simulink library "MOST_Lib"  (R2020b)
settings: Solver=VariableStepAuto  StartTime=0.0  StopTime=10.0  RelTol=1e-3
undefined symbols (not defined in the file or in its folder): simu, wind, windTurbine, windturbine, X, Y, Z
libraries: sm_lib x34, nesl_utility x28, $bdroot x6, simulink_extras x5, simulink x4, WECSim_Lib_Body_Elements x1
(part 1 of 2)
settings Model: VariantFading=off  Creator=kmruehl  LastModifiedBy=emili

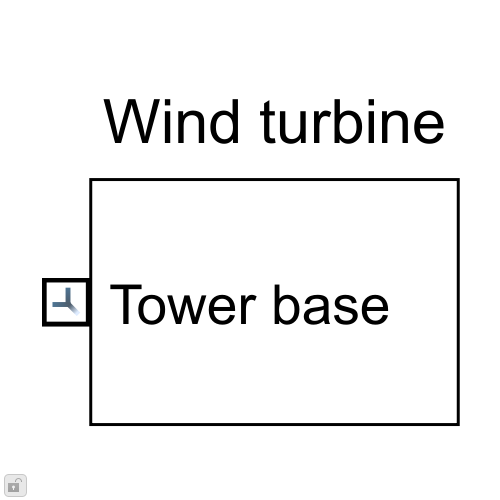
[thumbnail: Simulink's stored preview of the top-level diagram]
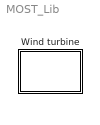
== system MOST_Lib ==
  Wind turbine : Subsystem  VariantControl=sv_1_IEA15MW  mask{windTurbine=windTurbine(1)}
      mask prompts: windTurbine "Wind Number:"
      mask init:
      | h1=[gcb '/Tower/Body Properties'];
      | geomFile=windTurbine.geometryFileTower;
      | set_param(h1,'ExtGeomFileName',geomFile);
      | geomType='STEP';
      | 
      | h1=[gcb '/Nacelle/Body Properties'];
      | geomFile=windTurbine.geometryFileNacelle;
      | set_param(h1,'ExtGeomFileName',geomFile);
      | geomType='STEP';
      | 
      | h1=[gcb '/Hub/Body Properties'];
      | geomFile=windTurbine.geometryFileHub;
      | set_param(h1,'ExtGeomFileName',geomFile);
      | geomType='STEP';
      | 
      | % h1=[gcb '/blade 1/Blade/Body Properties'];
      | % geomFile=windTurbine.geometryFileBlade;
      | % set_param(h1,'ExtGeomFileName',geomFile);
      | % geomType='STEP';
      | 
      | % h1=[gcb '/blade 2/Blade/Body Properties'];
      | % geomFile=windTurbine.geometryFileBlade;
      | % set_param(h1,'ExtGeomFileName',geomFile);
      | % geomType='STEP';
      | % 
      | % h1=[gcb '/blade 3/Blade/Body Properties'];
      | % geomFile=windTurbine.geometryFileBlade;
      | % set_param(h1,'ExtGeomFileName',geomFile);
      | % geomType='STEP';
      | 
      | % toWorkSpace: outputs
      | h=[gcb '/SignalOutput/To Workspace'];
      | responseName=['windTurbine' num2str(windTurbine.number) '_out'];
      | set_param(h,'VariableName',responseName);

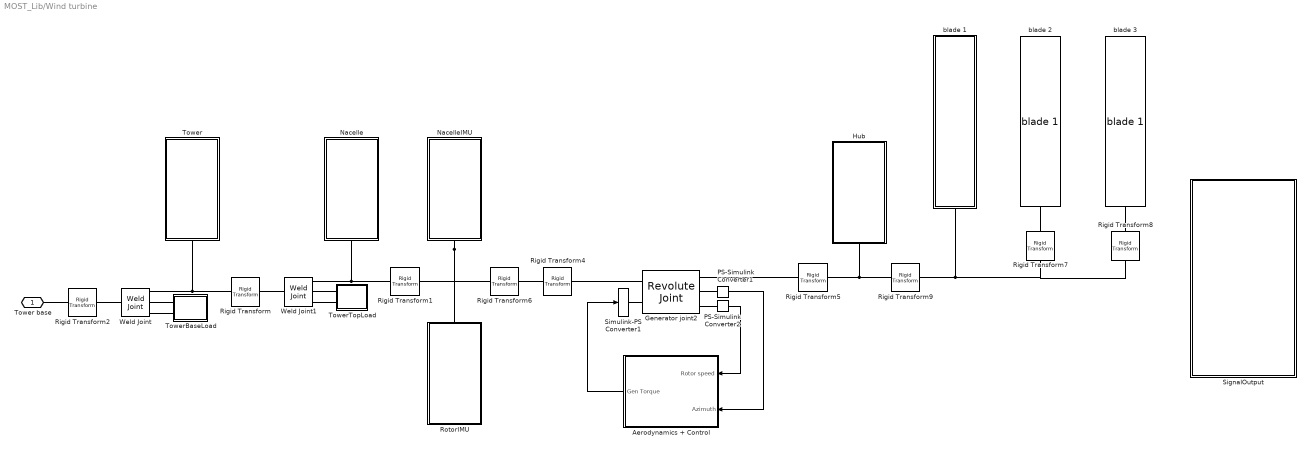
== system MOST_Lib/Wind turbine ==
  Aerodynamics + Control : Subsystem  VariantControl=sv_control2
  Generator joint2 : sm_lib/Joints/Revolute Joint  JointMode=Normal  SenseConstraintForce=off  SenseConstraintForceX=off  SenseConstraintForceY=off  SenseConstraintForceZ=off  SenseConstraintTorque=off  SenseConstraintTorqueX=off  SenseConstraintTorqueY=off  SenseConstraintTorqueZ=off  SenseTotalForce=off  SenseTotalForceX=off  SenseTotalForceY=off  SenseTotalForceZ=off  SenseTotalTorque=off  SenseTotalTorqueX=off  SenseTotalTorqueY=off  SenseTotalTorqueZ=off  CompositeWrenchDir=FollowerOnBase  CompositeWrenchFrame=BaseFrame  EquilibriumPosition=0  EquilibriumPositionUnits=deg  SpringStiffness=0  SpringStiffnessUnits=N*m/deg  DampingCoefficient=0  DampingCoefficientUnits=N*m/(deg/s)  TorqueActuationMode=InputTorque  MotionActuationMode=ComputedMotion  SensePosition=on  SenseVelocity=on  SenseAcceleration=off  SenseTorqueForce=off  SenseLowerLimitTorqueForce=off  SenseUpperLimitTorqueForce=off  LowerLimitSpecify=off  LowerLimitBound=-90  LowerLimitBoundUnits=deg  LowerLimitStiffness=1e4  LowerLimitStiffnessUnits=N*m/deg  LowerLimitDamping=10  LowerLimitDampingUnits=N*m/(deg/s)  LowerLimitTransitionRegionWidth=0.1  LowerLimitTransitionRegionWidthUnits=deg  UpperLimitSpecify=off  UpperLimitBound=90  UpperLimitBoundUnits=deg  UpperLimitStiffness=1e4  UpperLimitStiffnessUnits=N*m/deg  UpperLimitDamping=10  UpperLimitDampingUnits=N*m/(deg/s)  UpperLimitTransitionRegionWidth=0.1  UpperLimitTransitionRegionWidthUnits=deg  PositionTargetSpecify=on  PositionTargetPriority=High  PositionTargetValue=0  PositionTargetValueUnits=deg  VelocityTargetSpecify=on  VelocityTargetPriority=High  VelocityTargetValue=windTurbine.omega0  VelocityTargetValueUnits=rad/s
  Hub : Subsystem
  Nacelle : Subsystem
  NacelleIMU : Subsystem
  PS-Simulink Converter1 : nesl_utility/PS-Simulink Converter  PhysicalDomain=network_engine_domain  SubClassName=ps_output  LeftPortType=input  RightPortType=output  PseudoPeriodic=off  Frequencies=[]  VectorFormat=1-D array  Unit=rad  AffineConversion=off
  PS-Simulink Converter2 : nesl_utility/PS-Simulink Converter  PhysicalDomain=network_engine_domain  SubClassName=ps_output  LeftPortType=input  RightPortType=output  PseudoPeriodic=off  Frequencies=[]  VectorFormat=1-D array  Unit=rad/s  AffineConversion=off
  Rigid Transform : sm_lib/Frames and Transforms/Rigid Transform  TranslationMethod=StandardAxis  TranslationStandardOffset=windTurbine.tower.height  TranslationLengthUnit=m  TranslationStandardAxis=+Z  TranslationCartesianOffset=[0 0 0]  TranslationROffset=0  TranslationROffsetUnits=m  TranslationZOffset=0  TranslationZOffsetUnits=m  TranslationThetaOffset=0  TranslationThetaOffsetUnits=deg  RotationMethod=None  RotationAngleUnits=deg  RotationStandardAxis=+Z  RotationAngle=0.0  RotationArbitraryAxis=[0 0 1]  FollAlignAxisA=+X  BaseAlignAxisA=+Y  FollAlignAxisB=+Y  BaseAlignAxisB=+Z  RotationSequenceAxes=FollowerAxes  RotationSequence=XYX  RotationSequenceAngles=[0 0 0]  RotationSequenceAnglesUnits=deg  RotationMatrix=[1 0 0; 0 1 0; 0 0 1]  Quaternion=[1 0 0 0]
  Rigid Transform1 : sm_lib/Frames and Transforms/Rigid Transform  TranslationMethod=StandardAxis  TranslationStandardOffset=windTurbine.nacelle.Twr2Shft  TranslationLengthUnit=m  TranslationStandardAxis=+Z  TranslationCartesianOffset=[0 0 0]  TranslationROffset=0  TranslationROffsetUnits=m  TranslationZOffset=0  TranslationZOffsetUnits=m  TranslationThetaOffset=0  TranslationThetaOffsetUnits=deg  RotationMethod=None  RotationAngleUnits=deg  RotationStandardAxis=+Z  RotationAngle=0.0  RotationArbitraryAxis=[0 0 1]  FollAlignAxisA=+X  BaseAlignAxisA=+Y  FollAlignAxisB=+Y  BaseAlignAxisB=+Z  RotationSequenceAxes=FollowerAxes  RotationSequence=XYX  RotationSequenceAngles=[0 0 0]  RotationSequenceAnglesUnits=deg  RotationMatrix=[1 0 0; 0 1 0; 0 0 1]  Quaternion=[1 0 0 0]
  Rigid Transform2 : sm_lib/Frames and Transforms/Rigid Transform  TranslationMethod=StandardAxis  TranslationStandardOffset=windTurbine.tower.offset  TranslationLengthUnit=m  TranslationStandardAxis=+Z  TranslationCartesianOffset=[0 0 0]  TranslationROffset=0  TranslationROffsetUnits=m  TranslationZOffset=0  TranslationZOffsetUnits=m  TranslationThetaOffset=0  TranslationThetaOffsetUnits=deg  RotationMethod=None  RotationAngleUnits=deg  RotationStandardAxis=+Z  RotationAngle=0.0  RotationArbitraryAxis=[0 0 1]  FollAlignAxisA=+X  BaseAlignAxisA=+Y  FollAlignAxisB=+Y  BaseAlignAxisB=+Z  RotationSequenceAxes=FollowerAxes  RotationSequence=XYX  RotationSequenceAngles=[0 0 0]  RotationSequenceAnglesUnits=deg  RotationMatrix=[1 0 0; 0 1 0; 0 0 1]  Quaternion=[1 0 0 0]
  Rigid Transform4 : sm_lib/Frames and Transforms/Rigid Transform  TranslationMethod=None  TranslationStandardOffset=0  TranslationLengthUnit=m  TranslationStandardAxis=+Z  TranslationCartesianOffset=[0 0 0]  TranslationROffset=0  TranslationROffsetUnits=m  TranslationZOffset=0  TranslationZOffsetUnits=m  TranslationThetaOffset=0  TranslationThetaOffsetUnits=deg  RotationMethod=StandardAxis  RotationAngleUnits=deg  RotationStandardAxis=+Y  RotationAngle=90  RotationArbitraryAxis=[0 0 1]  FollAlignAxisA=+X  BaseAlignAxisA=+Y  FollAlignAxisB=+Y  BaseAlignAxisB=+Z  RotationSequenceAxes=FollowerAxes  RotationSequence=XYX  RotationSequenceAngles=[0 0 0]  RotationSequenceAnglesUnits=deg  RotationMatrix=[1 0 0; 0 1 0; 0 0 1]  Quaternion=[1 0 0 0]
  Rigid Transform5 : sm_lib/Frames and Transforms/Rigid Transform  TranslationMethod=None  TranslationStandardOffset=0  TranslationLengthUnit=m  TranslationStandardAxis=+Z  TranslationCartesianOffset=[0 0 0]  TranslationROffset=0  TranslationROffsetUnits=m  TranslationZOffset=0  TranslationZOffsetUnits=m  TranslationThetaOffset=0  TranslationThetaOffsetUnits=deg  RotationMethod=StandardAxis  RotationAngleUnits=deg  RotationStandardAxis=-Y  RotationAngle=90  RotationArbitraryAxis=[0 0 1]  FollAlignAxisA=+X  BaseAlignAxisA=+Y  FollAlignAxisB=+Y  BaseAlignAxisB=+Z  RotationSequenceAxes=FollowerAxes  RotationSequence=XYX  RotationSequenceAngles=[0 0 0]  RotationSequenceAnglesUnits=deg  RotationMatrix=[1 0 0; 0 1 0; 0 0 1]  Quaternion=[1 0 0 0]
  Rigid Transform6 : sm_lib/Frames and Transforms/Rigid Transform  TranslationMethod=None  TranslationStandardOffset=0  TranslationLengthUnit=m  TranslationStandardAxis=+Z  TranslationCartesianOffset=[0 0 0]  TranslationROffset=0  TranslationROffsetUnits=m  TranslationZOffset=0  TranslationZOffsetUnits=m  TranslationThetaOffset=0  TranslationThetaOffsetUnits=deg  RotationMethod=StandardAxis  RotationAngleUnits=deg  RotationStandardAxis=+Y  RotationAngle=windTurbine.nacelle.tiltangle  RotationArbitraryAxis=[0 0 1]  FollAlignAxisA=+X  BaseAlignAxisA=+Y  FollAlignAxisB=+Y  BaseAlignAxisB=+Z  RotationSequenceAxes=FollowerAxes  RotationSequence=XYX  RotationSequenceAngles=[0 0 0]  RotationSequenceAnglesUnits=deg  RotationMatrix=[1 0 0; 0 1 0; 0 0 1]  Quaternion=[1 0 0 0]
  Rigid Transform7 : sm_lib/Frames and Transforms/Rigid Transform  TranslationMethod=None  TranslationStandardOffset=0  TranslationLengthUnit=m  TranslationStandardAxis=+Z  TranslationCartesianOffset=[0 0 0]  TranslationROffset=0  TranslationROffsetUnits=m  TranslationZOffset=0  TranslationZOffsetUnits=m  TranslationThetaOffset=0  TranslationThetaOffsetUnits=deg  RotationMethod=StandardAxis  RotationAngleUnits=deg  RotationStandardAxis=+X  RotationAngle=120  RotationArbitraryAxis=[0 0 1]  FollAlignAxisA=+X  BaseAlignAxisA=+Y  FollAlignAxisB=+Y  BaseAlignAxisB=+Z  RotationSequenceAxes=FollowerAxes  RotationSequence=XYX  RotationSequenceAngles=[0 0 0]  RotationSequenceAnglesUnits=deg  RotationMatrix=[1 0 0; 0 1 0; 0 0 1]  Quaternion=[1 0 0 0]
  Rigid Transform8 : sm_lib/Frames and Transforms/Rigid Transform  TranslationMethod=None  TranslationStandardOffset=0  TranslationLengthUnit=m  TranslationStandardAxis=+Z  TranslationCartesianOffset=[0 0 0]  TranslationROffset=0  TranslationROffsetUnits=m  TranslationZOffset=0  TranslationZOffsetUnits=m  TranslationThetaOffset=0  TranslationThetaOffsetUnits=deg  RotationMethod=StandardAxis  RotationAngleUnits=deg  RotationStandardAxis=+X  RotationAngle=240  RotationArbitraryAxis=[0 0 1]  FollAlignAxisA=+X  BaseAlignAxisA=+Y  FollAlignAxisB=+Y  BaseAlignAxisB=+Z  RotationSequenceAxes=FollowerAxes  RotationSequence=XYX  RotationSequenceAngles=[0 0 0]  RotationSequenceAnglesUnits=deg  RotationMatrix=[1 0 0; 0 1 0; 0 0 1]  Quaternion=[1 0 0 0]
  Rigid Transform9 : sm_lib/Frames and Transforms/Rigid Transform  TranslationMethod=StandardAxis  TranslationStandardOffset=windTurbine.hub.overhang  TranslationLengthUnit=m  TranslationStandardAxis=+X  TranslationCartesianOffset=[0 0 0]  TranslationROffset=0  TranslationROffsetUnits=m  TranslationZOffset=0  TranslationZOffsetUnits=m  TranslationThetaOffset=0  TranslationThetaOffsetUnits=deg  RotationMethod=None  RotationAngleUnits=deg  RotationStandardAxis=+Z  RotationAngle=0.0  RotationArbitraryAxis=[0 0 1]  FollAlignAxisA=+X  BaseAlignAxisA=+Y  FollAlignAxisB=+Y  BaseAlignAxisB=+Z  RotationSequenceAxes=FollowerAxes  RotationSequence=XYX  RotationSequenceAngles=[0 0 0]  RotationSequenceAnglesUnits=deg  RotationMatrix=[1 0 0; 0 1 0; 0 0 1]  Quaternion=[1 0 0 0]
  RotorIMU : Subsystem
  SignalOutput : Subsystem
  Simulink-PS Converter1 : nesl_utility/Simulink-PS Converter  PhysicalDomain=network_engine_domain  SubClassName=ps_input  LeftPortType=input  RightPortType=output  PseudoPeriodic=off  Frequencies=[]  NoiseDistribution=none  NoiseParameters=[]  Unit=N*m  AffineConversion=off  FilteringAndDerivatives=provide  SimscapeFilterOrder=2  InputFilterTimeConstant=0.1  UdotUserProvided=0
  Tower : Subsystem
  TowerBaseLoad : Subsystem
  TowerTopLoad : Subsystem
  Weld Joint : sm_lib/Joints/Weld Joint  JointMode=Normal  SenseConstraintForce=on  SenseConstraintForceX=off  SenseConstraintForceY=off  SenseConstraintForceZ=off  SenseConstraintTorque=on  SenseConstraintTorqueX=off  SenseConstraintTorqueY=off  SenseConstraintTorqueZ=off  SenseTotalForce=off  SenseTotalForceX=off  SenseTotalForceY=off  SenseTotalForceZ=off  SenseTotalTorque=off  SenseTotalTorqueX=off  SenseTotalTorqueY=off  SenseTotalTorqueZ=off  CompositeWrenchDir=FollowerOnBase  CompositeWrenchFrame=BaseFrame
  Weld Joint1 : sm_lib/Joints/Weld Joint  JointMode=Normal  SenseConstraintForce=on  SenseConstraintForceX=off  SenseConstraintForceY=off  SenseConstraintForceZ=off  SenseConstraintTorque=on  SenseConstraintTorqueX=off  SenseConstraintTorqueY=off  SenseConstraintTorqueZ=off  SenseTotalForce=off  SenseTotalForceX=off  SenseTotalForceY=off  SenseTotalForceZ=off  SenseTotalTorque=off  SenseTotalTorqueX=off  SenseTotalTorqueY=off  SenseTotalTorqueZ=off  CompositeWrenchDir=FollowerOnBase  CompositeWrenchFrame=BaseFrame
  blade 1 : Subsystem  mask{bladeNumber=1, windTurbine=windTurbine}
      mask prompts: bladeNumber "Blade Number:"; windTurbine "windTurbine Number:"
      mask init:
      | h=[gcb '/bladeRootLoad/Goto'];
      |     responseName=['blade' num2str(bladeNumber) 'RootLoad'];
      |     set_param(h,'GotoTag',responseName);
      | 
      |     if exist('windTurbine','var') && ~isempty(windTurbine)
      |         h1=[gcb '/Blade/Body Properties'];
      |         geomFile=windTurbine.geometryFileBlade;
      |         set_param(h1,'ExtGeomFileName',geomFile);
      |     
      |         % mass=windTurbine.blade.mass;
      |         % set_param(h1,'Mass',mass)
      |         % 
      |         % inertia=windTurbine.blade.inertia;
      |         % set_param(h1,'MomentsOfInertia',inertia)
      |     end
  blade 2 : $bdroot/Wind turbine/blade 1  bladeNumber=2
  blade 3 : $bdroot/Wind turbine/blade 1  bladeNumber=3
  Tower base : PMIOPort  Side=Right
  wires:
  Aerodynamics + Control/1(Gen Torque) -> Simulink-PS Converter1/1
  PS-Simulink Converter1/1 -> Aerodynamics + Control/2(Azimuth)
  PS-Simulink Converter2/1 -> Aerodynamics + Control/1(" Rotor speed")
  connections:
  Nacelle/LConn1 -- Rigid Transform1/LConn1 -- Weld Joint1/RConn1
  blade 2/LConn1 -- Rigid Transform7/RConn1
  NacelleIMU/LConn1 -- Rigid Transform1/RConn1 -- RotorIMU/LConn1 -- Rigid Transform6/LConn1
  Hub/LConn1 -- Rigid Transform9/LConn1 -- Rigid Transform5/RConn1
  Tower/LConn1 -- Rigid Transform/LConn1 -- Weld Joint/RConn1
  Rigid Transform6/RConn1 -- Rigid Transform4/LConn1
  Rigid Transform4/RConn1 -- Generator joint2/LConn1
  Rigid Transform/RConn1 -- Weld Joint1/LConn1
  Rigid Transform2/LConn1 -- Tower base/RConn1
  PS-Simulink Converter2/LConn1 -- Generator joint2/RConn3
  Generator joint2/RConn1 -- Rigid Transform5/LConn1
  Rigid Transform2/RConn1 -- Weld Joint/LConn1
  Simulink-PS Converter1/RConn1 -- Generator joint2/LConn2
  PS-Simulink Converter1/LConn1 -- Generator joint2/RConn2
  Rigid Transform9/RConn1 -- Rigid Transform8/LConn1 -- Rigid Transform7/LConn1 -- blade 1/LConn1
  blade 3/LConn1 -- Rigid Transform8/RConn1
  Weld Joint/RConn2 -- TowerBaseLoad/LConn1
  Weld Joint/RConn3 -- TowerBaseLoad/LConn2
  Weld Joint1/RConn2 -- TowerTopLoad/LConn1
  Weld Joint1/RConn3 -- TowerTopLoad/LConn2

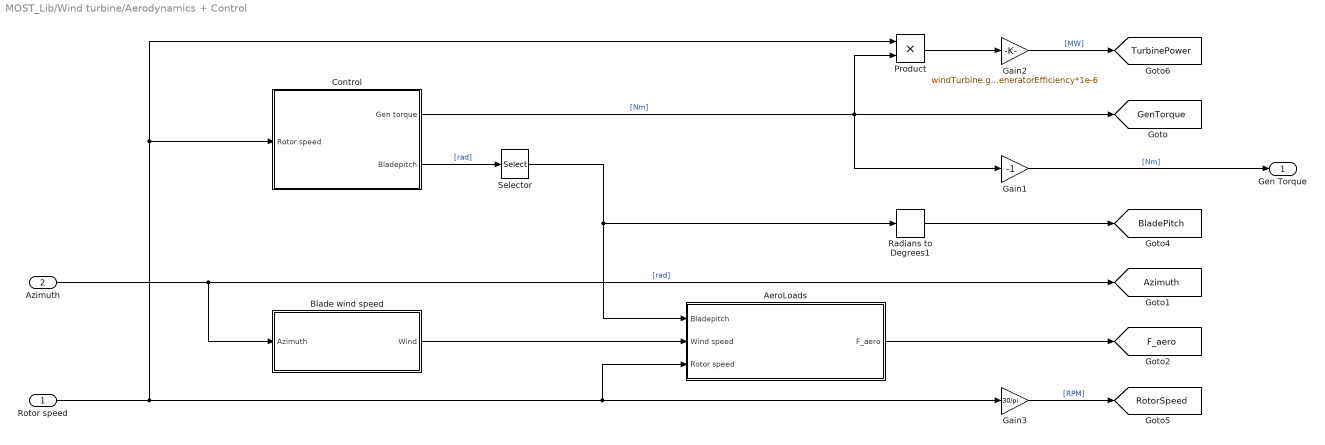
== system MOST_Lib/Wind turbine/Aerodynamics + Control ==
  " Rotor speed" : Inport
  Azimuth : Inport  Port=2
  AeroLoads : Subsystem
  Blade wind speed : Subsystem  Variant=on
  Control : Subsystem  Variant=on  VariantControl=sv_IEA10MW  mask{windturbine=windTurbine, wind=wind(1)}
      mask init:
      | h = [gcb,'/Baseline'];
      | sv1 = ['sv_' num2str(windturbine.number) '_control1'];
      | set_param(h,'VariantControl',sv1);
      | 
      | h = [gcb,'/ROSCO'];
      | sv2 = ['sv_' num2str(windturbine.number) '_control2'];
      | set_param(h,'VariantControl',sv2);
  Gain1 : Gain  Gain=-1
  Gain2 : Gain  Gain=windTurbine.generatorEfficiency*1e-6
  Gain3 : Gain  Gain=30/pi
  Product : Product
  Radians to Degrees1 : simulink_extras/Transformations/Radians to Degrees
  Selector : Selector  InputPortWidth=3  IndexOptions=Index vector (dialog)  Indices=1  OutputSizes=1
  Gen Torque : Outport  VectorParamsAs1DForOutWhenUnconnected=off
  tag scope: Azimuth=global, BladePitch=global, F_aero=global, GenTorque=global, RotorSpeed=global, TurbinePower=global
  wires:
  " Rotor speed"/1 -> Gain3/1, AeroLoads/3(Rotor speed), Product/1, Control/1(Rotor speed)
  Control/2(Bladepitch) -> Selector/1  "[rad]"
  Control/1(Gen torque) -> Product/2, Gain1/1, [GenTorque]  "[Nm]"
  Product/1 -> Gain2/1
  Azimuth/1 -> Blade wind speed/1(Azimuth), [Azimuth]  "[rad]"
  Gain3/1 -> [RotorSpeed]  "[RPM]"
  Gain1/1 -> Gen Torque/1  "[Nm]"
  Gain2/1 -> [TurbinePower]  "[MW]"
  Blade wind speed/1(Wind) -> AeroLoads/2(Wind speed)
  AeroLoads/1(F_aero) -> [F_aero]
  Radians to Degrees1/1 -> [BladePitch]
  Selector/1 -> AeroLoads/1(Bladepitch), Radians to Degrees1/1

== system MOST_Lib/Wind turbine/Hub ==
  Body Properties : sm_lib/Body Elements/File Solid  ExtGeomFileName=geometry\IEA15MW_Hub.STEP  UnitType=Custom  ExtGeomFileUnits=m  StepReaderType=HEX  ExportConvexHullGeometry=off  InertiaType=Custom  Mass=windTurbine.hub.mass  MassUnits=kg  CenterOfMass=[0 0 0]  CenterOfMassUnits=m  MomentsOfInertia=windTurbine.hub.inertia  MomentsOfInertiaUnits=kg*m^2  ProductsOfInertia=windTurbine.hub.inertiaProducts  ProductsOfInertiaUnits=kg*m^2  BasedOnType=Density  Density=1000  DensityUnits=kg/(m^3)  DoExposeReferenceFrame=on  SerializedFrames=<Frames/>  GraphicType=FromGeometry  MarkerShape=Sphere  MarkerSize=10  MarkerSizeUnits=m  GraphicVisPropType=AdvancedVisualProperties  GraphicDiffuseColor=[1 1 1]  GraphicSpecularColor=[0.5 0.5 0.5 1.0]  GraphicAmbientColor=[0.15 0.15 0.15 1.0]  GraphicEmissiveColor=[0.0 0.0 0.0 1.0]  GraphicShininess=75  GraphicOpacity=1
  Constant : Constant  Value=-windTurbine.hub.mass*simu.gravity
  External Force and Torque : sm_lib/Forces and Torques/External Force and Torque  ForceResolutionFrame=World  EnableForceX=off  EnableForceY=off  EnableForceZ=on  EnableForce=off  TorqueResolutionFrame=AttachedFrame  EnableTorqueX=off  EnableTorqueY=off  EnableTorqueZ=off  EnableTorque=off
  Rigid Transform (Setup CG) : sm_lib/Frames and Transforms/Rigid Transform  TranslationMethod=StandardAxis  TranslationStandardOffset=windTurbine.hub.overhang  TranslationLengthUnit=m  TranslationStandardAxis=+X  TranslationCartesianOffset=windTurbine.hub.overhang  TranslationROffset=0  TranslationROffsetUnits=m  TranslationZOffset=0  TranslationZOffsetUnits=m  TranslationThetaOffset=0  TranslationThetaOffsetUnits=deg  RotationMethod=None  RotationAngleUnits=rad  RotationStandardAxis=+Y  RotationAngle=0.05  RotationArbitraryAxis=[0.57735026918962584 0.57735026918962584 0.57735026918962584]  FollAlignAxisA=+X  BaseAlignAxisA=+Y  FollAlignAxisB=+Y  BaseAlignAxisB=+Z  RotationSequenceAxes=FollowerAxes  RotationSequence=XYX  RotationSequenceAngles=[0 0 0]  RotationSequenceAnglesUnits=deg  RotationMatrix=[1 0 0; 0 1 0; 0 0 1]  Quaternion=[1 0 0 0]
  Simulink-PS Converter : nesl_utility/Simulink-PS Converter  PhysicalDomain=network_engine_domain  SubClassName=ps_input  LeftPortType=input  RightPortType=output  PseudoPeriodic=off  Frequencies=[]  NoiseDistribution=none  NoiseParameters=[]  Unit=1  AffineConversion=off  FilteringAndDerivatives=provide  SimscapeFilterOrder=1  InputFilterTimeConstant=0.001  UdotUserProvided=0
  Conn1 : PMIOPort  Side=Left
  wires:
  Constant/1 -> Simulink-PS Converter/1
  connections:
  Rigid Transform (Setup CG)/RConn1 -- Body Properties/RConn1 -- External Force and Torque/RConn1
  Rigid Transform (Setup CG)/LConn1 -- Conn1/RConn1
  Simulink-PS Converter/RConn1 -- External Force and Torque/LConn1

== system MOST_Lib/Wind turbine/Nacelle ==
  Body Properties : sm_lib/Body Elements/File Solid  ExtGeomFileName=geometry\IEA15MW_Nacelle.STEP  UnitType=Custom  ExtGeomFileUnits=m  StepReaderType=HEX  ExportConvexHullGeometry=off  InertiaType=Custom  Mass=windTurbine.nacelle.mass  MassUnits=kg  CenterOfMass=[0 0 0]  CenterOfMassUnits=m  MomentsOfInertia=windTurbine.nacelle.inertia  MomentsOfInertiaUnits=kg*m^2  ProductsOfInertia=windTurbine.nacelle.inertiaProducts  ProductsOfInertiaUnits=kg*m^2  BasedOnType=Density  Density=1000  DensityUnits=kg/(m^3)  DoExposeReferenceFrame=on  SerializedFrames=<Frames/>  GraphicType=FromGeometry  MarkerShape=Sphere  MarkerSize=10  MarkerSizeUnits=m  GraphicVisPropType=SimpleVisualProperties  GraphicDiffuseColor=[1 1 1]  GraphicSpecularColor=[0.5 0.5 0.5 1.0]  GraphicAmbientColor=[0.15 0.15 0.15 1.0]  GraphicEmissiveColor=[0.0 0.0 0.0 1.0]  GraphicShininess=75  GraphicOpacity=1
  Constant : Constant  Value=-windTurbine.nacelle.mass*simu.gravity
  Constant1 : Constant  Value=-windTurbine.nacelle.mass_yawBearing*simu.gravity
  External Force and Torque : sm_lib/Forces and Torques/External Force and Torque  ForceResolutionFrame=World  EnableForceX=off  EnableForceY=off  EnableForceZ=on  EnableForce=off  TorqueResolutionFrame=AttachedFrame  EnableTorqueX=off  EnableTorqueY=off  EnableTorqueZ=off  EnableTorque=off
  External Force and Torque1 : sm_lib/Forces and Torques/External Force and Torque  ForceResolutionFrame=World  EnableForceX=off  EnableForceY=off  EnableForceZ=on  EnableForce=off  TorqueResolutionFrame=AttachedFrame  EnableTorqueX=off  EnableTorqueY=off  EnableTorqueZ=off  EnableTorque=off
  Inertia : sm_lib/Body Elements/Inertia  InertiaType=PointMass  Mass=windTurbine.nacelle.mass_yawBearing  MassUnits=kg  CenterOfMass=[0 0 0]  CenterOfMassUnits=m  MomentsOfInertia=[1 1 1]  MomentsOfInertiaUnits=kg*m^2  ProductsOfInertia=[0 0 0]  ProductsOfInertiaUnits=kg*m^2  GraphicType=Marker  MarkerShape=Inertia  MarkerSize=20  GraphicVisPropType=SimpleVisualProperties  GraphicDiffuseColor=[0.5 0.5 0.5]  GraphicSpecularColor=[0.5 0.5 0.5 1.0]  GraphicAmbientColor=[0.15 0.15 0.15 1.0]  GraphicEmissiveColor=[0.0 0.0 0.0 1.0]  GraphicShininess=75  GraphicOpacity=1.0
  Rigid Transform (Setup CG) : sm_lib/Frames and Transforms/Rigid Transform  TranslationMethod=Cartesian  TranslationStandardOffset=0  TranslationLengthUnit=m  TranslationStandardAxis=+Z  TranslationCartesianOffset=windTurbine.nacelle.cog_rel  TranslationROffset=0  TranslationROffsetUnits=m  TranslationZOffset=0  TranslationZOffsetUnits=m  TranslationThetaOffset=0  TranslationThetaOffsetUnits=deg  RotationMethod=None  RotationAngleUnits=rad  RotationStandardAxis=+Y  RotationAngle=0.05  RotationArbitraryAxis=[0.57735026918962584 0.57735026918962584 0.57735026918962584]  FollAlignAxisA=+X  BaseAlignAxisA=+Y  FollAlignAxisB=+Y  BaseAlignAxisB=+Z  RotationSequenceAxes=FollowerAxes  RotationSequence=XYX  RotationSequenceAngles=[0 0 0]  RotationSequenceAnglesUnits=deg  RotationMatrix=[1 0 0; 0 1 0; 0 0 1]  Quaternion=[1 0 0 0]
  Simulink-PS Converter : nesl_utility/Simulink-PS Converter  PhysicalDomain=network_engine_domain  SubClassName=ps_input  LeftPortType=input  RightPortType=output  PseudoPeriodic=off  Frequencies=[]  NoiseDistribution=none  NoiseParameters=[]  Unit=1  AffineConversion=off  FilteringAndDerivatives=provide  SimscapeFilterOrder=1  InputFilterTimeConstant=0.001  UdotUserProvided=0
  Simulink-PS Converter1 : nesl_utility/Simulink-PS Converter  PhysicalDomain=network_engine_domain  SubClassName=ps_input  LeftPortType=input  RightPortType=output  PseudoPeriodic=off  Frequencies=[]  NoiseDistribution=none  NoiseParameters=[]  Unit=1  AffineConversion=off  FilteringAndDerivatives=provide  SimscapeFilterOrder=1  InputFilterTimeConstant=0.001  UdotUserProvided=0
  Conn1 : PMIOPort  Side=Left
  wires:
  Constant/1 -> Simulink-PS Converter/1
  Constant1/1 -> Simulink-PS Converter1/1
  connections:
  Rigid Transform (Setup CG)/RConn1 -- Body Properties/RConn1 -- External Force and Torque/RConn1
  Inertia/RConn1 -- Rigid Transform (Setup CG)/LConn1 -- Conn1/RConn1 -- External Force and Torque1/RConn1
  Simulink-PS Converter/RConn1 -- External Force and Torque/LConn1
  Simulink-PS Converter1/RConn1 -- External Force and Torque1/LConn1

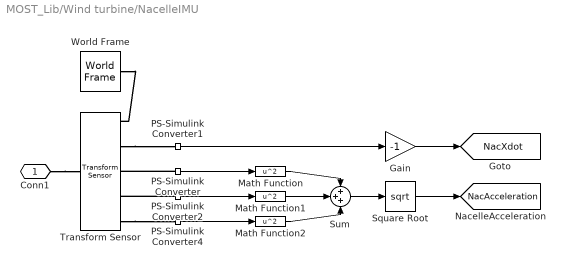
== system MOST_Lib/Wind turbine/NacelleIMU ==
  Gain : Gain  Gain=-1
  Math Function : Math  Operator=square  SaturateOnIntegerOverflow=on
  Math Function1 : Math  Operator=square  SaturateOnIntegerOverflow=on
  Math Function2 : Math  Operator=square  SaturateOnIntegerOverflow=on
  PS-Simulink Converter{,1..2,4} (4 alike) : nesl_utility/PS-Simulink Converter  PhysicalDomain=network_engine_domain  SubClassName=ps_output  LeftPortType=input  RightPortType=output  PseudoPeriodic=off  Frequencies=[]  VectorFormat=1-D array  Unit=inherit  AffineConversion=off
  Square Root : Sqrt  SaturateOnIntegerOverflow=on
  Sum : Sum  Inputs=+++
  Transform Sensor : sm_lib/Frames and Transforms/Transform Sensor  MeasurementFrame=World  SenseAngle=off  SenseAxis=off  SenseQ=off  SenseR=off  SenseRotationSequence=off  RotationSequence=XYX  SenseOmegaX=off  SenseOmegaY=off  SenseOmegaZ=off  SenseQDot=off  SenseRDot=off  SenseAlphaX=off  SenseAlphaY=off  SenseAlphaZ=off  SenseQDDot=off  SenseRDDot=off  SenseX=off  SenseY=off  SenseZ=off  SenseRad=off  SenseAzim=off  SenseDist=off  SenseInc=off  SenseXDot=on  SenseYDot=off  SenseZDot=off  SenseRadDot=off  SenseAzimDot=off  SenseDistDot=off  SenseIncDot=off  SenseXDDot=on  SenseYDDot=on  SenseZDDot=on  SenseRadDDot=off  SenseAzimDDot=off  SenseDistDDot=off  SenseIncDDot=off
  World Frame : sm_lib/Frames and Transforms/World Frame
  Conn1 : PMIOPort  Side=Left
  tag scope: NacAcceleration=global, NacXdot=global
  wires:
  PS-Simulink Converter1/1 -> Gain/1
  PS-Simulink Converter2/1 -> Math Function1/1
  Math Function2/1 -> Sum/3
  Math Function/1 -> Sum/1
  PS-Simulink Converter/1 -> Math Function/1
  Math Function1/1 -> Sum/2
  PS-Simulink Converter4/1 -> Math Function2/1
  Sum/1 -> Square Root/1
  Gain/1 -> [NacXdot]
  Square Root/1 -> [NacAcceleration]
  connections:
  Transform Sensor/RConn5 -- PS-Simulink Converter4/LConn1
  PS-Simulink Converter2/LConn1 -- Transform Sensor/RConn4
  PS-Simulink Converter/LConn1 -- Transform Sensor/RConn3
  Transform Sensor/RConn2 -- PS-Simulink Converter1/LConn1
  Conn1/RConn1 -- Transform Sensor/LConn1
  World Frame/RConn1 -- Transform Sensor/RConn1

== system MOST_Lib/Wind turbine/RotorIMU ==
  PS-Simulink Converter{,1,3..4} (4 alike) : nesl_utility/PS-Simulink Converter  PhysicalDomain=network_engine_domain  SubClassName=ps_output  LeftPortType=input  RightPortType=output  PseudoPeriodic=off  Frequencies=[]  VectorFormat=1-D array  Unit=inherit  AffineConversion=off
  Transform Sensor : sm_lib/Frames and Transforms/Transform Sensor  MeasurementFrame=World  SenseAngle=off  SenseAxis=off  SenseQ=on  SenseR=off  SenseRotationSequence=off  RotationSequence=XYX  SenseOmegaX=off  SenseOmegaY=on  SenseOmegaZ=on  SenseQDot=off  SenseRDot=off  SenseAlphaX=off  SenseAlphaY=off  SenseAlphaZ=off  SenseQDDot=off  SenseRDDot=off  SenseX=off  SenseY=off  SenseZ=off  SenseRad=off  SenseAzim=off  SenseDist=off  SenseInc=off  SenseXDot=on  SenseYDot=off  SenseZDot=off  SenseRadDot=off  SenseAzimDot=off  SenseDistDot=off  SenseIncDot=off  SenseXDDot=off  SenseYDDot=off  SenseZDDot=off  SenseRadDDot=off  SenseAzimDDot=off  SenseDistDDot=off  SenseIncDDot=off
  World Frame : sm_lib/Frames and Transforms/World Frame
  quaternion2EulXYZ : WECSim_Lib_Body_Elements/Rigid Body/Hydrodynamic Body/Structure/Motion Sensor/quaternion2EulXYZ  SystemSampleTime=-1  VariantActivationTime=update diagram  Latency=0  AutoFrameSizeCalculation=off
  Conn1 : PMIOPort  Side=Left
  tag scope: HUBpitch=global, HUBrot=global, HUBvx=global, HUByaw=global
  wires:
  PS-Simulink Converter4/1 -> [HUBvx]
  PS-Simulink Converter1/1 -> quaternion2EulXYZ/1
  PS-Simulink Converter/1 -> [HUBpitch]
  PS-Simulink Converter3/1 -> [HUByaw]
  quaternion2EulXYZ/1 -> [HUBrot]
  connections:
  PS-Simulink Converter1/LConn1 -- Transform Sensor/RConn2
  PS-Simulink Converter/LConn1 -- Transform Sensor/RConn3
  Transform Sensor/RConn4 -- PS-Simulink Converter3/LConn1
  Transform Sensor/RConn5 -- PS-Simulink Converter4/LConn1
  Transform Sensor/RConn1 -- Conn1/RConn1
  Transform Sensor/LConn1 -- World Frame/RConn1

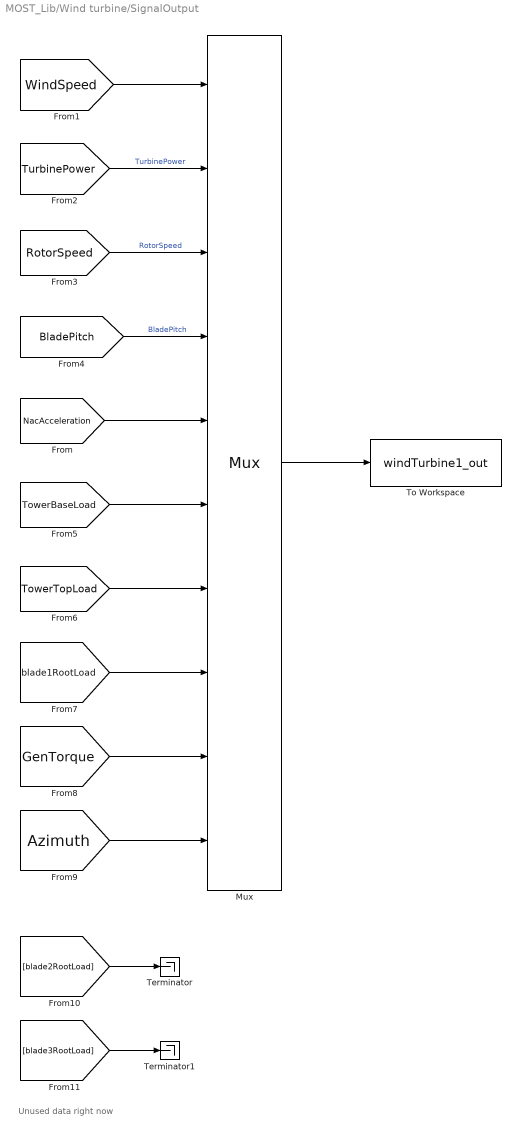
== system MOST_Lib/Wind turbine/SignalOutput ==
  note: Unused data right now
  Mux : Mux  Inputs=10
      settings: DisplayOption=signals
  Terminator : Terminator
  Terminator1 : Terminator
  To Workspace : ToWorkspace  VariableName=windTurbine1_out  SaveFormat=Structure With Time  SampleTime=simu.dtOut
  wires:
  Mux/1 -> To Workspace/1
  [blade2RootLoad] -> Terminator/1
  [blade3RootLoad] -> Terminator1/1
  [BladePitch] -> Mux/4  "BladePitch"
  [RotorSpeed] -> Mux/3  "RotorSpeed"
  [TurbinePower] -> Mux/2  "TurbinePower"
  [NacAcceleration] -> Mux/5
  [TowerBaseLoad] -> Mux/6
  [TowerTopLoad] -> Mux/7
  [blade1RootLoad] -> Mux/8
  [GenTorque] -> Mux/9
  [Azimuth] -> Mux/10
  [WindSpeed] -> Mux/1

== system MOST_Lib/Wind turbine/Tower ==
  Body Properties : sm_lib/Body Elements/File Solid  ExtGeomFileName=geometry\IEA15MW_Tower.STEP  UnitType=Custom  ExtGeomFileUnits=m  StepReaderType=HEX  ExportConvexHullGeometry=off  InertiaType=Custom  Mass=windTurbine.tower.mass  MassUnits=kg  CenterOfMass=[0 0 0]  CenterOfMassUnits=m  MomentsOfInertia=windTurbine.tower.inertia  MomentsOfInertiaUnits=kg*m^2  ProductsOfInertia=windTurbine.tower.inertiaProducts  ProductsOfInertiaUnits=kg*m^2  BasedOnType=Density  Density=1000  DensityUnits=kg/(m^3)  DoExposeReferenceFrame=on  SerializedFrames=<Frames/>  GraphicType=FromGeometry  MarkerShape=Sphere  MarkerSize=10  MarkerSizeUnits=m  GraphicVisPropType=SimpleVisualProperties  GraphicDiffuseColor=[1 1 1]  GraphicSpecularColor=[0.5 0.5 0.5 1.0]  GraphicAmbientColor=[0.15 0.15 0.15 1.0]  GraphicEmissiveColor=[0.0 0.0 0.0 1.0]  GraphicShininess=75  GraphicOpacity=1
  Constant : Constant  Value=-windTurbine.tower.mass*simu.gravity
  External Force and Torque : sm_lib/Forces and Torques/External Force and Torque  ForceResolutionFrame=World  EnableForceX=off  EnableForceY=off  EnableForceZ=on  EnableForce=off  TorqueResolutionFrame=AttachedFrame  EnableTorqueX=off  EnableTorqueY=off  EnableTorqueZ=off  EnableTorque=off
  Rigid Transform (Setup CG) : sm_lib/Frames and Transforms/Rigid Transform  TranslationMethod=Cartesian  TranslationStandardOffset=0  TranslationLengthUnit=m  TranslationStandardAxis=+Z  TranslationCartesianOffset=windTurbine.tower.cog_rel  TranslationROffset=0  TranslationROffsetUnits=m  TranslationZOffset=0  TranslationZOffsetUnits=m  TranslationThetaOffset=0  TranslationThetaOffsetUnits=deg  RotationMethod=None  RotationAngleUnits=rad  RotationStandardAxis=+Y  RotationAngle=0.05  RotationArbitraryAxis=[0.57735026918962584 0.57735026918962584 0.57735026918962584]  FollAlignAxisA=+X  BaseAlignAxisA=+Y  FollAlignAxisB=+Y  BaseAlignAxisB=+Z  RotationSequenceAxes=FollowerAxes  RotationSequence=XYX  RotationSequenceAngles=[0 0 0]  RotationSequenceAnglesUnits=deg  RotationMatrix=[1 0 0; 0 1 0; 0 0 1]  Quaternion=[1 0 0 0]
  Simulink-PS Converter : nesl_utility/Simulink-PS Converter  PhysicalDomain=network_engine_domain  SubClassName=ps_input  LeftPortType=input  RightPortType=output  PseudoPeriodic=off  Frequencies=[]  NoiseDistribution=none  NoiseParameters=[]  Unit=1  AffineConversion=off  FilteringAndDerivatives=provide  SimscapeFilterOrder=1  InputFilterTimeConstant=0.001  UdotUserProvided=0
  Conn1 : PMIOPort  Side=Left
  wires:
  Constant/1 -> Simulink-PS Converter/1
  connections:
  Simulink-PS Converter/RConn1 -- External Force and Torque/LConn1
  Rigid Transform (Setup CG)/LConn1 -- Conn1/RConn1
  Body Properties/RConn1 -- External Force and Torque/RConn1 -- Rigid Transform (Setup CG)/RConn1

== system MOST_Lib/Wind turbine/TowerBaseLoad ==
  Mux : Mux  Inputs=2
  PS-Simulink Converter6 : nesl_utility/PS-Simulink Converter  PhysicalDomain=network_engine_domain  SubClassName=ps_output  LeftPortType=input  RightPortType=output  PseudoPeriodic=off  Frequencies=[]  VectorFormat=1-D array  Unit=inherit  AffineConversion=off
  PS-Simulink Converter7 : nesl_utility/PS-Simulink Converter  PhysicalDomain=network_engine_domain  SubClassName=ps_output  LeftPortType=input  RightPortType=output  PseudoPeriodic=off  Frequencies=[]  VectorFormat=1-D array  Unit=inherit  AffineConversion=off
  fc : PMIOPort  Side=Left
  tc : PMIOPort  Port=2  Side=Left
  tag scope: TowerBaseLoad=global
  wires:
  PS-Simulink Converter7/1 -> Mux/1
  PS-Simulink Converter6/1 -> Mux/2
  Mux/1 -> [TowerBaseLoad]
  connections:
  fc/RConn1 -- PS-Simulink Converter7/LConn1
  tc/RConn1 -- PS-Simulink Converter6/LConn1

== system MOST_Lib/Wind turbine/TowerTopLoad ==
  Mux1 : Mux  Inputs=2
  PS-Simulink Converter8 : nesl_utility/PS-Simulink Converter  PhysicalDomain=network_engine_domain  SubClassName=ps_output  LeftPortType=input  RightPortType=output  PseudoPeriodic=off  Frequencies=[]  VectorFormat=1-D array  Unit=inherit  AffineConversion=off
  PS-Simulink Converter9 : nesl_utility/PS-Simulink Converter  PhysicalDomain=network_engine_domain  SubClassName=ps_output  LeftPortType=input  RightPortType=output  PseudoPeriodic=off  Frequencies=[]  VectorFormat=1-D array  Unit=inherit  AffineConversion=off
  fc : PMIOPort  Side=Left
  tc : PMIOPort  Port=2  Side=Left
  tag scope: TowerTopLoad=global
  wires:
  PS-Simulink Converter9/1 -> Mux1/1
  PS-Simulink Converter8/1 -> Mux1/2
  Mux1/1 -> [TowerTopLoad]
  connections:
  fc/RConn1 -- PS-Simulink Converter9/LConn1
  tc/RConn1 -- PS-Simulink Converter8/LConn1

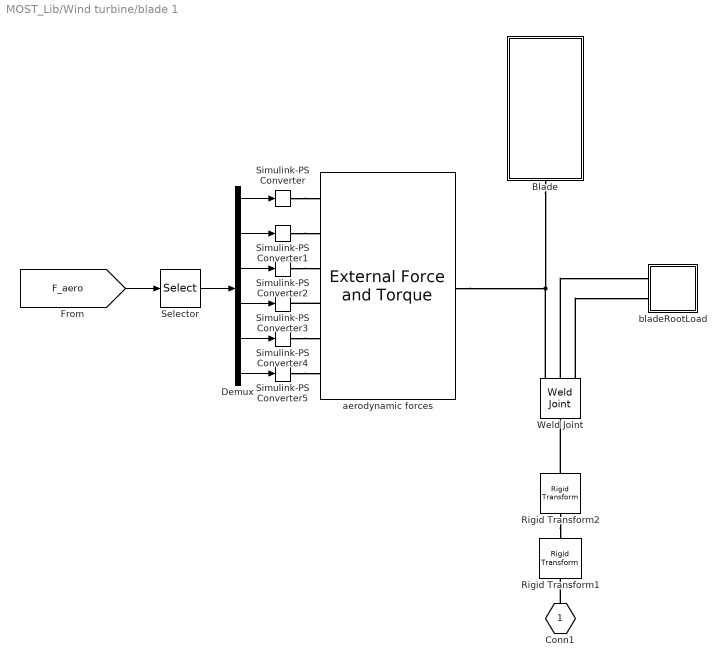
== system MOST_Lib/Wind turbine/blade 1 ==
  Blade : Subsystem
  Demux : Demux  Outputs=6
  Rigid Transform1 : sm_lib/Frames and Transforms/Rigid Transform  TranslationMethod=None  TranslationStandardOffset=0  TranslationLengthUnit=m  TranslationStandardAxis=+Z  TranslationCartesianOffset=[0 0 0]  TranslationROffset=0  TranslationROffsetUnits=m  TranslationZOffset=0  TranslationZOffsetUnits=m  TranslationThetaOffset=0  TranslationThetaOffsetUnits=deg  RotationMethod=StandardAxis  RotationAngleUnits=deg  RotationStandardAxis=-Y  RotationAngle=windTurbine.hub.precone  RotationArbitraryAxis=[0 0 1]  FollAlignAxisA=+X  BaseAlignAxisA=+Y  FollAlignAxisB=+Y  BaseAlignAxisB=+Z  RotationSequenceAxes=FollowerAxes  RotationSequence=XYX  RotationSequenceAngles=[0 0 0]  RotationSequenceAnglesUnits=deg  RotationMatrix=[1 0 0; 0 1 0; 0 0 1]  Quaternion=[1 0 0 0]
  Rigid Transform2 : sm_lib/Frames and Transforms/Rigid Transform  TranslationMethod=StandardAxis  TranslationStandardOffset=windTurbine.hub.Rhub  TranslationLengthUnit=m  TranslationStandardAxis=+Z  TranslationCartesianOffset=[0 0 0]  TranslationROffset=0  TranslationROffsetUnits=m  TranslationZOffset=0  TranslationZOffsetUnits=m  TranslationThetaOffset=0  TranslationThetaOffsetUnits=deg  RotationMethod=None  RotationAngleUnits=deg  RotationStandardAxis=+Z  RotationAngle=0.0  RotationArbitraryAxis=[0 0 1]  FollAlignAxisA=+X  BaseAlignAxisA=+Y  FollAlignAxisB=+Y  BaseAlignAxisB=+Z  RotationSequenceAxes=FollowerAxes  RotationSequence=XYX  RotationSequenceAngles=[0 0 0]  RotationSequenceAnglesUnits=deg  RotationMatrix=[1 0 0; 0 1 0; 0 0 1]  Quaternion=[1 0 0 0]
  Selector : Selector  NumberOfDimensions=2  InputPortWidth=3  IndexOptions=Select all,Index vector (dialog)  Indices=[1 3],bladeNumber  OutputSizes=1,1
  Simulink-PS Converter{,1..5} (6 alike) : nesl_utility/Simulink-PS Converter  PhysicalDomain=network_engine_domain  SubClassName=ps_input  LeftPortType=input  RightPortType=output  PseudoPeriodic=off  Frequencies=[]  NoiseDistribution=none  NoiseParameters=[]  Unit=1  AffineConversion=off  FilteringAndDerivatives=provide  SimscapeFilterOrder=1  InputFilterTimeConstant=0.001  UdotUserProvided=0
  Weld Joint : sm_lib/Joints/Weld Joint  JointMode=Normal  SenseConstraintForce=on  SenseConstraintForceX=off  SenseConstraintForceY=off  SenseConstraintForceZ=off  SenseConstraintTorque=on  SenseConstraintTorqueX=off  SenseConstraintTorqueY=off  SenseConstraintTorqueZ=off  SenseTotalForce=off  SenseTotalForceX=off  SenseTotalForceY=off  SenseTotalForceZ=off  SenseTotalTorque=off  SenseTotalTorqueX=off  SenseTotalTorqueY=off  SenseTotalTorqueZ=off  CompositeWrenchDir=FollowerOnBase  CompositeWrenchFrame=BaseFrame
  aerodynamic forces : sm_lib/Forces and Torques/External Force and Torque  ForceResolutionFrame=AttachedFrame  EnableForceX=on  EnableForceY=on  EnableForceZ=on  EnableForce=off  TorqueResolutionFrame=AttachedFrame  EnableTorqueX=on  EnableTorqueY=on  EnableTorqueZ=on  EnableTorque=off
  bladeRootLoad : Subsystem
  Conn1 : PMIOPort  Side=Left
  wires:
  Demux/5 -> Simulink-PS Converter4/1
  Demux/3 -> Simulink-PS Converter2/1
  Demux/4 -> Simulink-PS Converter3/1
  Demux/6 -> Simulink-PS Converter5/1
  Demux/1 -> Simulink-PS Converter/1
  [F_aero] -> Selector/1
  Selector/1 -> Demux/1
  Demux/2 -> Simulink-PS Converter1/1
  connections:
  Rigid Transform2/LConn1 -- Rigid Transform1/RConn1
  Rigid Transform2/RConn1 -- Weld Joint/LConn1
  Conn1/RConn1 -- Rigid Transform1/LConn1
  Weld Joint/RConn1 -- aerodynamic forces/RConn1 -- Blade/LConn1
  Simulink-PS Converter4/RConn1 -- aerodynamic forces/LConn5
  Simulink-PS Converter1/RConn1 -- aerodynamic forces/LConn2
  Simulink-PS Converter5/RConn1 -- aerodynamic forces/LConn6
  aerodynamic forces/LConn4 -- Simulink-PS Converter3/RConn1
  Simulink-PS Converter/RConn1 -- aerodynamic forces/LConn1
  Simulink-PS Converter2/RConn1 -- aerodynamic forces/LConn3
  Weld Joint/RConn2 -- bladeRootLoad/LConn1
  Weld Joint/RConn3 -- bladeRootLoad/LConn2

== system MOST_Lib/Wind turbine/Aerodynamics + Control/AeroLoads ==
  Bladepitch : Inport
  Wind speed : Inport  Port=2
  Rotor speed : Inport  Port=3
  For Iterator Subsystem : Subsystem  TreatAsAtomicUnit=on
  F_aero : Outport  PortDimensions=[6 3]
  wires:
  Wind speed/1 -> For Iterator Subsystem/1(Wind speed)
  Rotor speed/1 -> For Iterator Subsystem/2(Rotor speed)
  Bladepitch/1 -> For Iterator Subsystem/3(Bladepitch)
  For Iterator Subsystem/1(F_aero) -> F_aero/1

== system MOST_Lib/Wind turbine/Aerodynamics + Control/Blade wind speed ==
  note: 1) Add Subsystem or Model blocks as valid variant choices.
      | 2) You cannot connect blocks at this level. At simulation, connectivity is automatically
      | determined, based on the active variant and port name matching.
  Azimuth : Inport
  Constant wind : Subsystem  VariantControl=sv_wind_constant
  Turbulent wind : Subsystem  VariantControl=sv_wind_turbulent
  Wind : Outport

== system MOST_Lib/Wind turbine/Aerodynamics + Control/Control ==
  note: 1) Add Subsystem or Model blocks as valid variant choices.
      | 2) You cannot connect blocks at this level. At simulation, connectivity is automatically
      | determined, based on the active variant and port name matching.
  Rotor speed : Inport
  Baseline : Subsystem  VariantControl=sv_1_control1
  ROSCO : Subsystem  VariantControl=sv_1_control2
  Gen torque : Outport
  Bladepitch : Outport  Port=2

== system MOST_Lib/Wind turbine/blade 1/Blade ==
  Body Properties : sm_lib/Body Elements/File Solid  ExtGeomFileName=geometry\IEA15MW_Blade.STEP  UnitType=Custom  ExtGeomFileUnits=m  StepReaderType=HEX  ExportConvexHullGeometry=off  InertiaType=Custom  Mass=windTurbine.blade.mass  MassUnits=kg  CenterOfMass=[0 0 0]  CenterOfMassUnits=m  MomentsOfInertia=windTurbine.blade.inertia  MomentsOfInertiaUnits=kg*m^2  ProductsOfInertia=[0 0 0]  ProductsOfInertiaUnits=kg*m^2  BasedOnType=Density  Density=1000  DensityUnits=kg/(m^3)  DoExposeReferenceFrame=on  SerializedFrames=<Frames/>  GraphicType=FromGeometry  MarkerShape=Sphere  MarkerSize=10  MarkerSizeUnits=m  GraphicVisPropType=SimpleVisualProperties  GraphicDiffuseColor=[1 1 1]  GraphicSpecularColor=[0.5 0.5 0.5 1.0]  GraphicAmbientColor=[0.15 0.15 0.15 1.0]  GraphicEmissiveColor=[0.0 0.0 0.0 1.0]  GraphicShininess=75  GraphicOpacity=1
  Constant : Constant  Value=-windTurbine.blade.mass*simu.gravity
  External Force and Torque : sm_lib/Forces and Torques/External Force and Torque  ForceResolutionFrame=World  EnableForceX=off  EnableForceY=off  EnableForceZ=on  EnableForce=off  TorqueResolutionFrame=AttachedFrame  EnableTorqueX=off  EnableTorqueY=off  EnableTorqueZ=off  EnableTorque=off
  Rigid Transform (Setup CG) : sm_lib/Frames and Transforms/Rigid Transform  TranslationMethod=Cartesian  TranslationStandardOffset=0  TranslationLengthUnit=m  TranslationStandardAxis=+Z  TranslationCartesianOffset=windTurbine.blade.cog_rel  TranslationROffset=0  TranslationROffsetUnits=m  TranslationZOffset=0  TranslationZOffsetUnits=m  TranslationThetaOffset=0  TranslationThetaOffsetUnits=deg  RotationMethod=None  RotationAngleUnits=rad  RotationStandardAxis=+Y  RotationAngle=0.05  RotationArbitraryAxis=[0.57735026918962584 0.57735026918962584 0.57735026918962584]  FollAlignAxisA=+X  BaseAlignAxisA=+Y  FollAlignAxisB=+Y  BaseAlignAxisB=+Z  RotationSequenceAxes=FollowerAxes  RotationSequence=XYX  RotationSequenceAngles=[0 0 0]  RotationSequenceAnglesUnits=deg  RotationMatrix=[1 0 0; 0 1 0; 0 0 1]  Quaternion=[1 0 0 0]
  Simulink-PS Converter : nesl_utility/Simulink-PS Converter  PhysicalDomain=network_engine_domain  SubClassName=ps_input  LeftPortType=input  RightPortType=output  PseudoPeriodic=off  Frequencies=[]  NoiseDistribution=none  NoiseParameters=[]  Unit=1  AffineConversion=off  FilteringAndDerivatives=provide  SimscapeFilterOrder=1  InputFilterTimeConstant=0.001  UdotUserProvided=0
  Conn1 : PMIOPort  Side=Left
  wires:
  Constant/1 -> Simulink-PS Converter/1
  connections:
  Rigid Transform (Setup CG)/RConn1 -- Body Properties/RConn1 -- External Force and Torque/RConn1
  Rigid Transform (Setup CG)/LConn1 -- Conn1/RConn1
  Simulink-PS Converter/RConn1 -- External Force and Torque/LConn1

== system MOST_Lib/Wind turbine/blade 1/bladeRootLoad ==
  Mux : Mux  Inputs=2
  PS-Simulink Converter5 : nesl_utility/PS-Simulink Converter  PhysicalDomain=network_engine_domain  SubClassName=ps_output  LeftPortType=input  RightPortType=output  PseudoPeriodic=off  Frequencies=[]  VectorFormat=1-D array  Unit=inherit  AffineConversion=off
  PS-Simulink Converter6 : nesl_utility/PS-Simulink Converter  PhysicalDomain=network_engine_domain  SubClassName=ps_output  LeftPortType=input  RightPortType=output  PseudoPeriodic=off  Frequencies=[]  VectorFormat=1-D array  Unit=inherit  AffineConversion=off
  fc : PMIOPort  Side=Left
  tc : PMIOPort  Port=2  Side=Left
  tag scope: blade1RootLoad=global
  wires:
  PS-Simulink Converter6/1 -> Mux/2
  Mux/1 -> [blade1RootLoad]
  PS-Simulink Converter5/1 -> Mux/1
  connections:
  tc/RConn1 -- PS-Simulink Converter6/LConn1
  fc/RConn1 -- PS-Simulink Converter5/LConn1

== system MOST_Lib/Wind turbine/Aerodynamics + Control/AeroLoads/For Iterator Subsystem ==
  Wind speed : Inport
  Rotor speed : Inport  Port=2
  Bladepitch : Inport  Port=3
  " " : Subsystem
  For Iterator : ForIterator  IterationLimit=6
  F_aero : Outport
  wires:
  Wind speed/1 -> " "/1(Wind speed)
  Rotor speed/1 -> " "/2(Rotor speed)
  Bladepitch/1 -> " "/3(Bladepitch)
  " "/1(F_aero) -> F_aero/1
  For Iterator/1 -> " "/4(Index)

== system MOST_Lib/Wind turbine/Aerodynamics + Control/Blade wind speed/Constant wind ==
  Azimuth : Inport
  " " : Subsystem
  "   " : $bdroot/Wind turbine/Aerodynamics + Control/Blade wind speed/Constant wind/   SystemSampleTime=-1  VariantActivationTime=update diagram  Latency=0  AutoFrameSizeCalculation=off
  "     " : $bdroot/Wind turbine/Aerodynamics + Control/Blade wind speed/Constant wind/   SystemSampleTime=-1  VariantActivationTime=update diagram  Latency=0  AutoFrameSizeCalculation=off
  Bias : Bias  Bias=deg2rad(120)
  Bias1 : Bias  Bias=deg2rad(240)
  Constant2 : Constant  Value=wind.meanVelocity
  Mux : Mux  Inputs=3
  Wind : Outport
  tag scope: WindSpeed=global
  wires:
  Constant2/1 -> [WindSpeed]
  " "/1(Wind) -> Mux/1
  "     "/1 -> Mux/3
  "   "/1 -> Mux/2
  Bias1/1 -> "     "/1
  Mux/1 -> Wind/1  "Wind+Relative motion"
  Azimuth/1 -> " "/1(Azimuth), Bias/1, Bias1/1
  Bias/1 -> "   "/1

== system MOST_Lib/Wind turbine/Aerodynamics + Control/Blade wind speed/Turbulent wind ==
  Azimuth : Inport
  " " : Bias  Bias=deg2rad(120)
  "  " : Bias  Bias=deg2rad(240)
  "   " : Subsystem
  "       " : $bdroot/Wind turbine/Aerodynamics + Control/Blade wind speed/Turbulent wind/     SystemSampleTime=-1  VariantActivationTime=update diagram  Latency=0  AutoFrameSizeCalculation=off
  "        " : $bdroot/Wind turbine/Aerodynamics + Control/Blade wind speed/Turbulent wind/     SystemSampleTime=-1  VariantActivationTime=update diagram  Latency=0  AutoFrameSizeCalculation=off
  Clock : Clock
  Constant : Constant  Value=0
  Constant1 : Constant  Value=windTurbine.hub.height
  Mux : Mux  Inputs=3
  Wind Table : Lookup_n-D  NumberOfTableDimensions=3  BreakpointsForDimension1=wind.time  BreakpointsForDimension2=wind.yDiscr  BreakpointsForDimension3=wind.zDiscr  BeginIndexSearchUsingPreviousIndexResult=on  Table=wind.velocity  InternalRulePriority=Speed  RndMeth=Simplest  InputSameDT=on
      settings: InputPortMap=u0,u1,u2
  Wind : Outport
  tag scope: WindSpeed=global
  wires:
  Wind Table/1 -> [WindSpeed]
  Clock/1 -> Wind Table/1
  "   "/1(wind) -> Mux/1
  "        "/1 -> Mux/3
  Constant1/1 -> Wind Table/3
  Constant/1 -> Wind Table/2
  "       "/1 -> Mux/2
  "  "/1 -> "        "/1
  Mux/1 -> Wind/1  "Wind + Relative motion"
  Azimuth/1 -> "   "/1(azimuth), " "/1, "  "/1
  " "/1 -> "       "/1

== system MOST_Lib/Wind turbine/Aerodynamics + Control/Control/Baseline ==
  Rotor speed : Inport
  " " : StateSpace  A=windTurbine.Baseline.omegaFilter.A  B=windTurbine.Baseline.omegaFilter.B  C=windTurbine.Baseline.omegaFilter.C  D=windTurbine.Baseline.omegaFilter.D  InitialCondition=[0,windTurbine.omega0/windTurbine.Baseline.omegaFilter.C(2)]
  Bladepitch control : Subsystem
  Torque control : Lookup_n-D  NumberOfTableDimensions=1  BreakpointsForDimension1=windTurbine.Baseline.omega_gen  Table=windTurbine.Baseline.Cgen  RndMeth=Simplest  InputSameDT=on
  Gen torque : Outport
  Bladepitch : Outport  Port=2
  wires:
  " "/1 -> Torque control/1, Bladepitch control/1(Rotor speed)
  Rotor speed/1 -> " "/1
  Torque control/1 -> Gen torque/1  "Nm"
  Bladepitch control/1(Bladepitch) -> Bladepitch/1  "rad"

== system MOST_Lib/Wind turbine/Aerodynamics + Control/Control/ROSCO ==
  Rotor speed : Inport
  "ROSCO controller " : Subsystem
  Gen torque : Outport
  Bladepitch : Outport  Port=2
  wires:
  Rotor speed/1 -> "ROSCO controller "/1(Generator speed)  "rad/s"
  [NacXdot] -> "ROSCO controller "/3(NacXdot)  "m/s"
  "ROSCO controller "/2(Bladepitch) -> Bladepitch/1  "rad"
  [WindSpeed] -> "ROSCO controller "/2(Wind speed)  "m/s"
  "ROSCO controller "/1(Gen torque) -> Gen torque/1  "Nm"
